simulink subsystem "ButtonStatesRF"  (R2023b) (continued)
== system ButtonStatesRF/ButtonStates/fnCallSubsystem/Function-Call Subsystem23 == (continued)

== system ButtonStatesRF/ButtonStates/fnCallSubsystem/Function-Call Subsystem24 ==
  BLU_FnClkIncDateDig3 : TriggerPort  TriggerType=function-call  IsSimulinkFunction=on  FunctionName=BLU_FnClkIncDateDig3  VariantControl=(inherit)

== system ButtonStatesRF/ButtonStates/fnCallSubsystem/Function-Call Subsystem25 ==
  BLU_FnClkIncDateDig2 : TriggerPort  TriggerType=function-call  IsSimulinkFunction=on  FunctionName=BLU_FnClkIncDateDig2  VariantControl=(inherit)

== system ButtonStatesRF/ButtonStates/fnCallSubsystem/Function-Call Subsystem26 ==
  BLU_FnClkIncDateDig1 : TriggerPort  TriggerType=function-call  IsSimulinkFunction=on  FunctionName=BLU_FnClkIncDateDig1  VariantControl=(inherit)

== system ButtonStatesRF/ButtonStates/fnCallSubsystem/Function-Call Subsystem27 ==
  BLU_FnClkDecDateDig3 : TriggerPort  TriggerType=function-call  IsSimulinkFunction=on  FunctionName=BLU_FnClkDecDateDig3  VariantControl=(inherit)

== system ButtonStatesRF/ButtonStates/fnCallSubsystem/Function-Call Subsystem28 ==
  BLU_FnClkDecDateDig2 : TriggerPort  TriggerType=function-call  IsSimulinkFunction=on  FunctionName=BLU_FnClkDecDateDig2  VariantControl=(inherit)

== system ButtonStatesRF/ButtonStates/fnCallSubsystem/Function-Call Subsystem29 ==
  BLU_FnClkDecDateDig1 : TriggerPort  TriggerType=function-call  IsSimulinkFunction=on  FunctionName=BLU_FnClkDecDateDig1  VariantControl=(inherit)

== system ButtonStatesRF/ButtonStates/fnCallSubsystem/Function-Call Subsystem3 ==
  BLU_FnRLResetRndTmr : TriggerPort  TriggerType=function-call  IsSimulinkFunction=on  FunctionName=BLU_FnRLResetRndTmr  VariantControl=(inherit)

== system ButtonStatesRF/ButtonStates/fnCallSubsystem/Function-Call Subsystem30 ==
  BLU_FnSetHeatedGrips : TriggerPort  TriggerType=function-call  IsSimulinkFunction=on  FunctionName=BLU_FnSetHeatedGrips  VariantControl=(inherit)
  u : ArgIn  OutDataTypeStr=Enum: eStateName  ArgumentName=u
  HeatedGripsOut : Outport
  wires:
  u/1 -> HeatedGripsOut/1

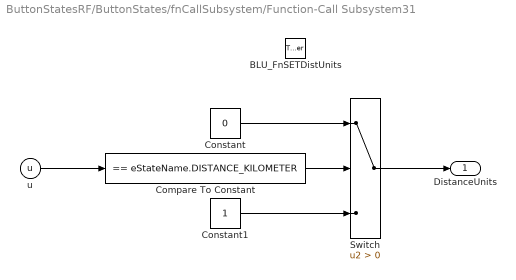
== system ButtonStatesRF/ButtonStates/fnCallSubsystem/Function-Call Subsystem31 ==
  BLU_FnSETDistUnits : TriggerPort  TriggerType=function-call  IsSimulinkFunction=on  FunctionName=BLU_FnSETDistUnits  VariantControl=(inherit)
  Compare To Constant : simulink/Logic and Bit Operations/Compare To Constant  relop===  const=eStateName.DISTANCE_KILOMETER  OutDataTypeStr=boolean
  Constant : Constant  Value=0  OutDataTypeStr=uint8
  Constant1 : Constant  OutDataTypeStr=uint8
  Switch : Switch  Criteria=u2 > Threshold  SaturateOnIntegerOverflow=off  InputSameDT=off
  u : ArgIn  OutDataTypeStr=Enum: eStateName  ArgumentName=u
  DistanceUnits : Outport
  wires:
  Constant1/1 -> Switch/3
  Constant/1 -> Switch/1
  Compare To Constant/1 -> Switch/2
  u/1 -> Compare To Constant/1
  Switch/1 -> DistanceUnits/1

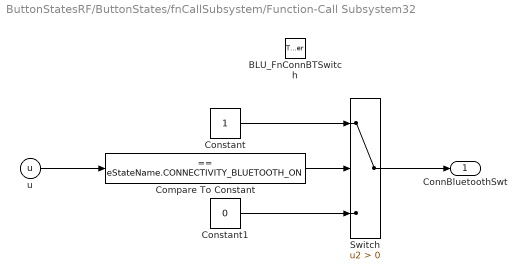
== system ButtonStatesRF/ButtonStates/fnCallSubsystem/Function-Call Subsystem32 ==
  BLU_FnConnBTSwitch : TriggerPort  TriggerType=function-call  IsSimulinkFunction=on  FunctionName=BLU_FnConnBTSwitch  VariantControl=(inherit)
  Compare To Constant : simulink/Logic and Bit Operations/Compare To Constant  relop===  const=eStateName.CONNECTIVITY_BLUETOOTH_ON  OutDataTypeStr=boolean
  Constant : Constant  OutDataTypeStr=uint8
  Constant1 : Constant  Value=0  OutDataTypeStr=uint8
  Switch : Switch  Criteria=u2 > Threshold  SaturateOnIntegerOverflow=off  InputSameDT=off
  u : ArgIn  OutDataTypeStr=Enum: eStateName  ArgumentName=u
  ConnBluetoothSwt : Outport
  wires:
  Switch/1 -> ConnBluetoothSwt/1
  u/1 -> Compare To Constant/1
  Compare To Constant/1 -> Switch/2
  Constant/1 -> Switch/1
  Constant1/1 -> Switch/3

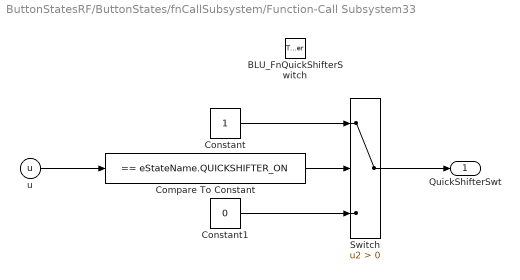
== system ButtonStatesRF/ButtonStates/fnCallSubsystem/Function-Call Subsystem33 ==
  BLU_FnQuickShifterSwitch : TriggerPort  TriggerType=function-call  IsSimulinkFunction=on  FunctionName=BLU_FnQuickShifterSwitch  VariantControl=(inherit)
  Compare To Constant : simulink/Logic and Bit Operations/Compare To Constant  relop===  const=eStateName.QUICKSHIFTER_ON  OutDataTypeStr=boolean
  Constant : Constant  OutDataTypeStr=uint8
  Constant1 : Constant  Value=0  OutDataTypeStr=uint8
  Switch : Switch  Criteria=u2 > Threshold  SaturateOnIntegerOverflow=off  InputSameDT=off
  u : ArgIn  OutDataTypeStr=Enum: eStateName  ArgumentName=u
  QuickShifterSwt : Outport
  wires:
  Constant1/1 -> Switch/3
  Constant/1 -> Switch/1
  Compare To Constant/1 -> Switch/2
  u/1 -> Compare To Constant/1
  Switch/1 -> QuickShifterSwt/1

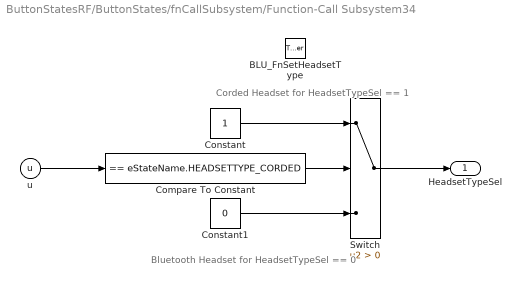
== system ButtonStatesRF/ButtonStates/fnCallSubsystem/Function-Call Subsystem34 ==
  note: Corded Headset for HeadsetTypeSel == 1
  note: Bluetooth Headset for HeadsetTypeSel == 0
  BLU_FnSetHeadsetType : TriggerPort  TriggerType=function-call  IsSimulinkFunction=on  FunctionName=BLU_FnSetHeadsetType  VariantControl=(inherit)
  Compare To Constant : simulink/Logic and Bit Operations/Compare To Constant  relop===  const=eStateName.HEADSETTYPE_CORDED  OutDataTypeStr=boolean
  Constant : Constant  OutDataTypeStr=uint8
  Constant1 : Constant  Value=0  OutDataTypeStr=uint8
  Switch : Switch  Criteria=u2 > Threshold  SaturateOnIntegerOverflow=off  InputSameDT=off
  u : ArgIn  OutDataTypeStr=Enum: eStateName  ArgumentName=u
  HeadsetTypeSel : Outport
  wires:
  Switch/1 -> HeadsetTypeSel/1
  u/1 -> Compare To Constant/1
  Compare To Constant/1 -> Switch/2
  Constant/1 -> Switch/1
  Constant1/1 -> Switch/3

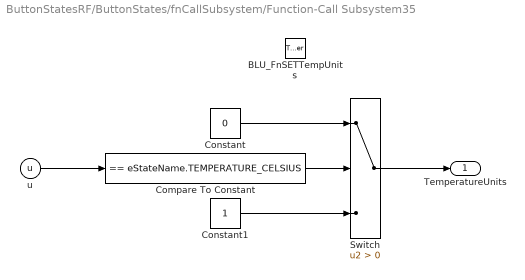
== system ButtonStatesRF/ButtonStates/fnCallSubsystem/Function-Call Subsystem35 ==
  BLU_FnSETTempUnits : TriggerPort  TriggerType=function-call  IsSimulinkFunction=on  FunctionName=BLU_FnSETTempUnits  VariantControl=(inherit)
  Compare To Constant : simulink/Logic and Bit Operations/Compare To Constant  relop===  const=eStateName.TEMPERATURE_CELSIUS  OutDataTypeStr=boolean
  Constant : Constant  Value=0  OutDataTypeStr=uint8
  Constant1 : Constant  OutDataTypeStr=uint8
  Switch : Switch  Criteria=u2 > Threshold  SaturateOnIntegerOverflow=off  InputSameDT=off
  u : ArgIn  OutDataTypeStr=Enum: eStateName  ArgumentName=u
  TemperatureUnits : Outport
  wires:
  Switch/1 -> TemperatureUnits/1
  u/1 -> Compare To Constant/1
  Compare To Constant/1 -> Switch/2
  Constant/1 -> Switch/1
  Constant1/1 -> Switch/3

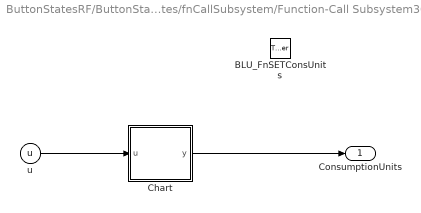
== system ButtonStatesRF/ButtonStates/fnCallSubsystem/Function-Call Subsystem36 ==
  BLU_FnSETConsUnits : TriggerPort  TriggerType=function-call  IsSimulinkFunction=on  FunctionName=BLU_FnSETConsUnits  VariantControl=(inherit)
  Chart : Stateflow chart  TreatAsAtomicUnit=on
  u : ArgIn  OutDataTypeStr=Enum: eStateName  ArgumentName=u
  ConsumptionUnits : Outport
  wires:
  u/1 -> Chart/1(u)
  Chart/1(y) -> ConsumptionUnits/1

== system ButtonStatesRF/ButtonStates/fnCallSubsystem/Function-Call Subsystem37 ==
  BLU_FnIncSrvcDistKM : TriggerPort  TriggerType=function-call  IsSimulinkFunction=on  FunctionName=BLU_FnIncSrvcDistKM  VariantControl=(inherit)

== system ButtonStatesRF/ButtonStates/fnCallSubsystem/Function-Call Subsystem38 ==
  BLU_FnDecSrvcDistKM : TriggerPort  TriggerType=function-call  IsSimulinkFunction=on  FunctionName=BLU_FnDecSrvcDistKM  VariantControl=(inherit)

== system ButtonStatesRF/ButtonStates/fnCallSubsystem/Function-Call Subsystem39 ==
  BLU_FnIncSrvcDistMI : TriggerPort  TriggerType=function-call  IsSimulinkFunction=on  FunctionName=BLU_FnIncSrvcDistMI  VariantControl=(inherit)

== system ButtonStatesRF/ButtonStates/fnCallSubsystem/Function-Call Subsystem4 ==
  BLU_FnTIResetTrip1 : TriggerPort  TriggerType=function-call  IsSimulinkFunction=on  FunctionName=BLU_FnTIResetTrip1  VariantControl=(inherit)

== system ButtonStatesRF/ButtonStates/fnCallSubsystem/Function-Call Subsystem40 ==
  BLU_FnDecSrvcDistMI : TriggerPort  TriggerType=function-call  IsSimulinkFunction=on  FunctionName=BLU_FnDecSrvcDistMI  VariantControl=(inherit)

== system ButtonStatesRF/ButtonStates/fnCallSubsystem/Function-Call Subsystem41 ==
  BLU_FnDecSrvcMonths : TriggerPort  TriggerType=function-call  IsSimulinkFunction=on  FunctionName=BLU_FnDecSrvcMonths  VariantControl=(inherit)

== system ButtonStatesRF/ButtonStates/fnCallSubsystem/Function-Call Subsystem42 ==
  BLU_FnIncSrvcMonths : TriggerPort  TriggerType=function-call  IsSimulinkFunction=on  FunctionName=BLU_FnIncSrvcMonths  VariantControl=(inherit)

== system ButtonStatesRF/ButtonStates/fnCallSubsystem/Function-Call Subsystem43 ==
  BLU_FnSaveSrvcChanges : TriggerPort  TriggerType=function-call  IsSimulinkFunction=on  FunctionName=BLU_FnSaveSrvcChanges  VariantControl=(inherit)

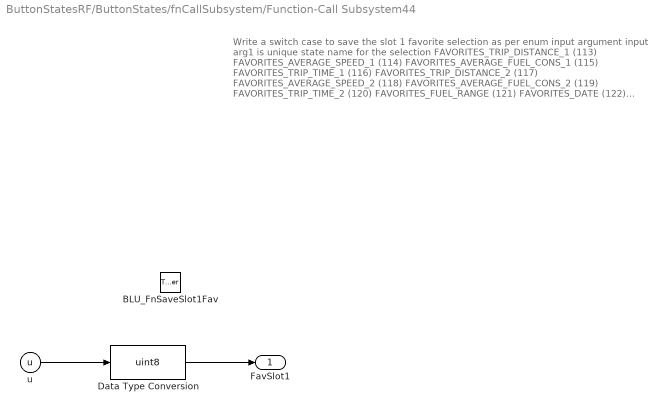
== system ButtonStatesRF/ButtonStates/fnCallSubsystem/Function-Call Subsystem44 ==
  note: Write a switch case to save the slot 1 favorite selection as per enum input argument
      | input arg1 is unique state name for the selection
      | FAVORITES_TRIP_DISTANCE_1 (113)
      | FAVORITES_AVERAGE_SPEED_1 (114)
      | FAVORITES_AVERAGE_FUEL_CONS_1 (115)
      | FAVORITES_TRIP_TIME_1 (116)
      | FAVORITES_TRIP_DISTANCE_2 (117)
      | FAVORITES_AVERAGE_SPEED_2 (118)
      | FAVORITES_AVERAGE_FUEL_CONS_2 (119)
      | FAVORITES_TRIP_TIME_2 (120)
      | FAVORITES_FUEL_RANGE (121)
      | FAVORITES_DATE (122)
      | FAVORITES_BATTERY (123)
      | FAVORITES_WATER_TEMPERATURE (124)
      | FAVORITES_ODOMETER (125)
      | FAVORITES_SLOT_1 (126)
      | FAVORITES_SLOT_2 (127)
      | FAVORITES_SLOT_3 (128)
      | FAVORITES_SLOT_4 (129)
  BLU_FnSaveSlot1Fav : TriggerPort  TriggerType=function-call  IsSimulinkFunction=on  FunctionName=BLU_FnSaveSlot1Fav  VariantControl=(inherit)
  Data Type Conversion : DataTypeConversion  OutDataTypeStr=uint8  SaturateOnIntegerOverflow=off  RndMeth=Floor
  u : ArgIn  ArgumentName=u
  FavSlot1 : Outport  OutDataTypeStr=uint8
  wires:
  u/1 -> Data Type Conversion/1
  Data Type Conversion/1 -> FavSlot1/1

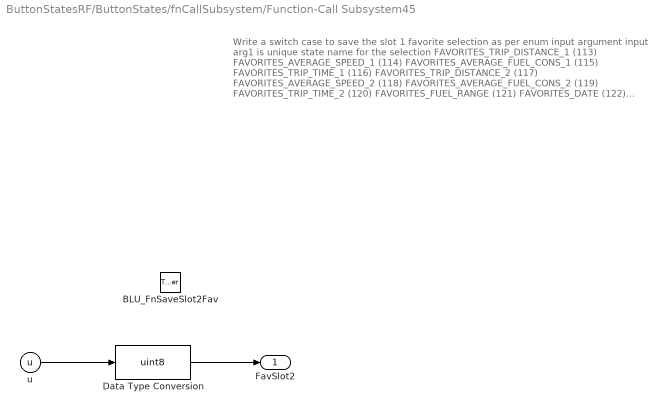
== system ButtonStatesRF/ButtonStates/fnCallSubsystem/Function-Call Subsystem45 ==
  note: Write a switch case to save the slot 1 favorite selection as per enum input argument
      | input arg1 is unique state name for the selection
      | FAVORITES_TRIP_DISTANCE_1 (113)
      | FAVORITES_AVERAGE_SPEED_1 (114)
      | FAVORITES_AVERAGE_FUEL_CONS_1 (115)
      | FAVORITES_TRIP_TIME_1 (116)
      | FAVORITES_TRIP_DISTANCE_2 (117)
      | FAVORITES_AVERAGE_SPEED_2 (118)
      | FAVORITES_AVERAGE_FUEL_CONS_2 (119)
      | FAVORITES_TRIP_TIME_2 (120)
      | FAVORITES_FUEL_RANGE (121)
      | FAVORITES_DATE (122)
      | FAVORITES_BATTERY (123)
      | FAVORITES_WATER_TEMPERATURE (124)
      | FAVORITES_ODOMETER (125)
      | FAVORITES_SLOT_1 (126)
      | FAVORITES_SLOT_2 (127)
      | FAVORITES_SLOT_3 (128)
      | FAVORITES_SLOT_4 (129)
  BLU_FnSaveSlot2Fav : TriggerPort  TriggerType=function-call  IsSimulinkFunction=on  FunctionName=BLU_FnSaveSlot2Fav  VariantControl=(inherit)
  Data Type Conversion : DataTypeConversion  OutDataTypeStr=uint8  SaturateOnIntegerOverflow=off  RndMeth=Floor
  u : ArgIn  ArgumentName=u
  FavSlot2 : Outport  OutDataTypeStr=uint8
  wires:
  u/1 -> Data Type Conversion/1
  Data Type Conversion/1 -> FavSlot2/1

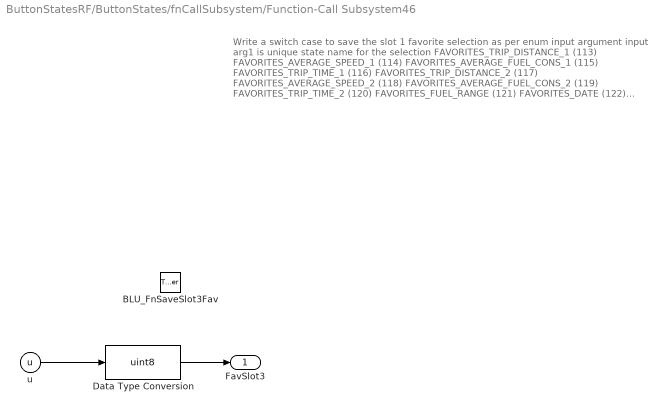
== system ButtonStatesRF/ButtonStates/fnCallSubsystem/Function-Call Subsystem46 ==
  note: Write a switch case to save the slot 1 favorite selection as per enum input argument
      | input arg1 is unique state name for the selection
      | FAVORITES_TRIP_DISTANCE_1 (113)
      | FAVORITES_AVERAGE_SPEED_1 (114)
      | FAVORITES_AVERAGE_FUEL_CONS_1 (115)
      | FAVORITES_TRIP_TIME_1 (116)
      | FAVORITES_TRIP_DISTANCE_2 (117)
      | FAVORITES_AVERAGE_SPEED_2 (118)
      | FAVORITES_AVERAGE_FUEL_CONS_2 (119)
      | FAVORITES_TRIP_TIME_2 (120)
      | FAVORITES_FUEL_RANGE (121)
      | FAVORITES_DATE (122)
      | FAVORITES_BATTERY (123)
      | FAVORITES_WATER_TEMPERATURE (124)
      | FAVORITES_ODOMETER (125)
      | FAVORITES_SLOT_1 (126)
      | FAVORITES_SLOT_2 (127)
      | FAVORITES_SLOT_3 (128)
      | FAVORITES_SLOT_4 (129)
  BLU_FnSaveSlot3Fav : TriggerPort  TriggerType=function-call  IsSimulinkFunction=on  FunctionName=BLU_FnSaveSlot3Fav  VariantControl=(inherit)
  Data Type Conversion : DataTypeConversion  OutDataTypeStr=uint8  SaturateOnIntegerOverflow=off  RndMeth=Floor
  u : ArgIn  ArgumentName=u
  FavSlot3 : Outport  OutDataTypeStr=uint8
  wires:
  u/1 -> Data Type Conversion/1
  Data Type Conversion/1 -> FavSlot3/1

== system ButtonStatesRF/ButtonStates/fnCallSubsystem/Function-Call Subsystem47 ==
  note: Write a switch case to save the slot 1 favorite selection as per enum input argument
      | input arg1 is unique state name for the selection
      | FAVORITES_TRIP_DISTANCE_1 (113)
      | FAVORITES_AVERAGE_SPEED_1 (114)
      | FAVORITES_AVERAGE_FUEL_CONS_1 (115)
      | FAVORITES_TRIP_TIME_1 (116)
      | FAVORITES_TRIP_DISTANCE_2 (117)
      | FAVORITES_AVERAGE_SPEED_2 (118)
      | FAVORITES_AVERAGE_FUEL_CONS_2 (119)
      | FAVORITES_TRIP_TIME_2 (120)
      | FAVORITES_FUEL_RANGE (121)
      | FAVORITES_DATE (122)
      | FAVORITES_BATTERY (123)
      | FAVORITES_WATER_TEMPERATURE (124)
      | FAVORITES_ODOMETER (125)
      | FAVORITES_SLOT_1 (126)
      | FAVORITES_SLOT_2 (127)
      | FAVORITES_SLOT_3 (128)
      | FAVORITES_SLOT_4 (129)
  BLU_FnSaveSlot4Fav : TriggerPort  TriggerType=function-call  IsSimulinkFunction=on  FunctionName=BLU_FnSaveSlot4Fav  VariantControl=(inherit)
  Data Type Conversion : DataTypeConversion  OutDataTypeStr=uint8  SaturateOnIntegerOverflow=off  RndMeth=Floor
  u : ArgIn  ArgumentName=u
  FavSlot4 : Outport  OutDataTypeStr=uint8
  wires:
  u/1 -> Data Type Conversion/1
  Data Type Conversion/1 -> FavSlot4/1

== system ButtonStatesRF/ButtonStates/fnCallSubsystem/Function-Call Subsystem48 ==
  BLU_FnLaunchControlON : TriggerPort  TriggerType=function-call  IsSimulinkFunction=on  FunctionName=BLU_FnLaunchControlON  VariantControl=(inherit)

== system ButtonStatesRF/ButtonStates/fnCallSubsystem/Function-Call Subsystem49 ==
  BLU_FnLaunchControlOFF : TriggerPort  TriggerType=function-call  IsSimulinkFunction=on  FunctionName=BLU_FnLaunchControlOFF  VariantControl=(inherit)

== system ButtonStatesRF/ButtonStates/fnCallSubsystem/Function-Call Subsystem5 ==
  BLU_FnTIResetTrip2 : TriggerPort  TriggerType=function-call  IsSimulinkFunction=on  FunctionName=BLU_FnTIResetTrip2  VariantControl=(inherit)

== system ButtonStatesRF/ButtonStates/fnCallSubsystem/Function-Call Subsystem50 ==
  BLU_FnShiftLightOFF : TriggerPort  TriggerType=function-call  IsSimulinkFunction=on  FunctionName=BLU_FnShiftLightOFF  VariantControl=(inherit)

== system ButtonStatesRF/ButtonStates/fnCallSubsystem/Function-Call Subsystem51 ==
  BLU_FnShiftLightON : TriggerPort  TriggerType=function-call  IsSimulinkFunction=on  FunctionName=BLU_FnShiftLightON  VariantControl=(inherit)

== system ButtonStatesRF/ButtonStates/fnCallSubsystem/Function-Call Subsystem52 ==
  BLU_FnSetQuickSelectorUP : TriggerPort  TriggerType=function-call  IsSimulinkFunction=on  FunctionName=BLU_FnSetQuickSelectorUP  VariantControl=(inherit)
  Terminator : Terminator
  u : ArgIn  ArgumentName=u
  wires:
  u/1 -> Terminator/1

== system ButtonStatesRF/ButtonStates/fnCallSubsystem/Function-Call Subsystem53 ==
  BLU_FnSetQuickSelectorDOWN : TriggerPort  TriggerType=function-call  IsSimulinkFunction=on  FunctionName=BLU_FnSetQuickSelectorDOWN  VariantControl=(inherit)
  Terminator : Terminator
  u : ArgIn  ArgumentName=u
  wires:
  u/1 -> Terminator/1

== system ButtonStatesRF/ButtonStates/fnCallSubsystem/Function-Call Subsystem54 ==
  BLU_FnNavigationStatus : TriggerPort  TriggerType=function-call  IsSimulinkFunction=on  FunctionName=BLU_FnNavigationStatus  VariantControl=(inherit)
  Terminator : Terminator
  u : ArgIn  ArgumentName=u
  wires:
  u/1 -> Terminator/1

== system ButtonStatesRF/ButtonStates/fnCallSubsystem/Function-Call Subsystem55 ==
  BLU_FnSETLanguage : TriggerPort  TriggerType=function-call  IsSimulinkFunction=on  FunctionName=BLU_FnSETLanguage  VariantControl=(inherit)
  Terminator : Terminator
  u : ArgIn  OutDataTypeStr=Enum: eStateName  ArgumentName=u
  wires:
  u/1 -> Terminator/1

== system ButtonStatesRF/ButtonStates/fnCallSubsystem/Function-Call Subsystem56 ==
  BLU_FnTISetAsOvwTrip1 : TriggerPort  TriggerType=function-call  IsSimulinkFunction=on  FunctionName=BLU_FnTISetAsOvwTrip1  VariantControl=(inherit)

== system ButtonStatesRF/ButtonStates/fnCallSubsystem/Function-Call Subsystem57 ==
  BLU_FnTISetAsOvwTrip2 : TriggerPort  TriggerType=function-call  IsSimulinkFunction=on  FunctionName=BLU_FnTISetAsOvwTrip2  VariantControl=(inherit)

== system ButtonStatesRF/ButtonStates/fnCallSubsystem/Function-Call Subsystem58 ==
  BLU_FnClkFrmtTgle : TriggerPort  TriggerType=function-call  IsSimulinkFunction=on  FunctionName=BLU_FnClkFrmtTgle  VariantControl=(inherit)
  Terminator : Terminator
  u : ArgIn  OutDataTypeStr=Enum: eStateName  ArgumentName=u
  wires:
  u/1 -> Terminator/1

== system ButtonStatesRF/ButtonStates/fnCallSubsystem/Function-Call Subsystem59 ==
  BLU_FnDateFrmtTgle : TriggerPort  TriggerType=function-call  IsSimulinkFunction=on  FunctionName=BLU_FnDateFrmtTgle  VariantControl=(inherit)
  Terminator : Terminator
  u : ArgIn  OutDataTypeStr=Enum: eStateName  ArgumentName=u
  wires:
  u/1 -> Terminator/1

== system ButtonStatesRF/ButtonStates/fnCallSubsystem/Function-Call Subsystem6 ==
  BLU_FnBIWarningsUp : TriggerPort  TriggerType=function-call  IsSimulinkFunction=on  FunctionName=BLU_FnBIWarningsUp  VariantControl=(inherit)

== system ButtonStatesRF/ButtonStates/fnCallSubsystem/Function-Call Subsystem60 ==
  BLU_FnSetHeatedGripsMode : TriggerPort  TriggerType=function-call  IsSimulinkFunction=on  FunctionName=BLU_FnSetHeatedGripsMode  VariantControl=(inherit)
  Terminator : Terminator
  u : ArgIn  OutDataTypeStr=Enum: eStateName  ArgumentName=u
  wires:
  u/1 -> Terminator/1

== system ButtonStatesRF/ButtonStates/fnCallSubsystem/Function-Call Subsystem61 ==
  BLU_FnIncomingCallAnswer : TriggerPort  TriggerType=function-call  IsSimulinkFunction=on  FunctionName=BLU_FnIncomingCallAnswer  VariantControl=(inherit)

== system ButtonStatesRF/ButtonStates/fnCallSubsystem/Function-Call Subsystem62 ==
  BLU_FnIncomingCallReject : TriggerPort  TriggerType=function-call  IsSimulinkFunction=on  FunctionName=BLU_FnIncomingCallReject  VariantControl=(inherit)

== system ButtonStatesRF/ButtonStates/fnCallSubsystem/Function-Call Subsystem63 ==
  BLU_FnCallVolInc : TriggerPort  TriggerType=function-call  IsSimulinkFunction=on  FunctionName=BLU_FnCallVolInc  VariantControl=(inherit)

== system ButtonStatesRF/ButtonStates/fnCallSubsystem/Function-Call Subsystem64 ==
  BLU_FnCallVolDec : TriggerPort  TriggerType=function-call  IsSimulinkFunction=on  FunctionName=BLU_FnCallVolDec  VariantControl=(inherit)

== system ButtonStatesRF/ButtonStates/fnCallSubsystem/Function-Call Subsystem65 ==
  BLU_FnEndCall : TriggerPort  TriggerType=function-call  IsSimulinkFunction=on  FunctionName=BLU_FnEndCall  VariantControl=(inherit)

== system ButtonStatesRF/ButtonStates/fnCallSubsystem/Function-Call Subsystem66 ==
  BLU_FnBTPhoneDvcUp : TriggerPort  TriggerType=function-call  IsSimulinkFunction=on  FunctionName=BLU_FnBTPhoneDvcUp  VariantControl=(inherit)

== system ButtonStatesRF/ButtonStates/fnCallSubsystem/Function-Call Subsystem67 ==
  BLU_FnBTPhoneDvcDwn : TriggerPort  TriggerType=function-call  IsSimulinkFunction=on  FunctionName=BLU_FnBTPhoneDvcDwn  VariantControl=(inherit)

== system ButtonStatesRF/ButtonStates/fnCallSubsystem/Function-Call Subsystem68 ==
  BLU_FnBTHeadsetDvcUp : TriggerPort  TriggerType=function-call  IsSimulinkFunction=on  FunctionName=BLU_FnBTHeadsetDvcUp  VariantControl=(inherit)

== system ButtonStatesRF/ButtonStates/fnCallSubsystem/Function-Call Subsystem69 ==
  BLU_FnBTHeadsetDvcDwn : TriggerPort  TriggerType=function-call  IsSimulinkFunction=on  FunctionName=BLU_FnBTHeadsetDvcDwn  VariantControl=(inherit)

== system ButtonStatesRF/ButtonStates/fnCallSubsystem/Function-Call Subsystem7 ==
  BLU_FnBIWarningsDwn : TriggerPort  TriggerType=function-call  IsSimulinkFunction=on  FunctionName=BLU_FnBIWarningsDwn  VariantControl=(inherit)

== system ButtonStatesRF/ButtonStates/fnCallSubsystem/Function-Call Subsystem70 ==
  BLU_FnBTPhoneDvcDel : TriggerPort  TriggerType=function-call  IsSimulinkFunction=on  FunctionName=BLU_FnBTPhoneDvcDel  VariantControl=(inherit)

== system ButtonStatesRF/ButtonStates/fnCallSubsystem/Function-Call Subsystem71 ==
  BLU_FnBTHeadsetDvcDel : TriggerPort  TriggerType=function-call  IsSimulinkFunction=on  FunctionName=BLU_FnBTHeadsetDvcDel  VariantControl=(inherit)

== system ButtonStatesRF/ButtonStates/fnCallSubsystem/Function-Call Subsystem72 ==
  BLU_FnBTPhonePassKeyChkd : TriggerPort  TriggerType=function-call  IsSimulinkFunction=on  FunctionName=BLU_FnBTPhonePassKeyChkd  VariantControl=(inherit)

== system ButtonStatesRF/ButtonStates/fnCallSubsystem/Function-Call Subsystem73 ==
  BLU_FnBTPhonePairReq : TriggerPort  TriggerType=function-call  IsSimulinkFunction=on  FunctionName=BLU_FnBTPhonePairReq  VariantControl=(inherit)

== system ButtonStatesRF/ButtonStates/fnCallSubsystem/Function-Call Subsystem74 ==
  BLU_FnBTHeadsetPairReq : TriggerPort  TriggerType=function-call  IsSimulinkFunction=on  FunctionName=BLU_FnBTHeadsetPairReq  VariantControl=(inherit)

== system ButtonStatesRF/ButtonStates/fnCallSubsystem/Function-Call Subsystem75 ==
  BLU_FnCnctvtyCnfrmAppReg : TriggerPort  TriggerType=function-call  IsSimulinkFunction=on  FunctionName=BLU_FnCnctvtyCnfrmAppReg  VariantControl=(inherit)
  Terminator : Terminator
  u : ArgIn  ArgumentName=u
  wires:
  u/1 -> Terminator/1

== system ButtonStatesRF/ButtonStates/fnCallSubsystem/Function-Call Subsystem76 ==
  BLU_FnHdstSelCord : TriggerPort  TriggerType=function-call  IsSimulinkFunction=on  FunctionName=BLU_FnHdstSelCord  VariantControl=(inherit)

== system ButtonStatesRF/ButtonStates/fnCallSubsystem/Function-Call Subsystem77 ==
  BLU_FnHdstSelBT : TriggerPort  TriggerType=function-call  IsSimulinkFunction=on  FunctionName=BLU_FnHdstSelBT  VariantControl=(inherit)

== system ButtonStatesRF/ButtonStates/fnCallSubsystem/Function-Call Subsystem78 ==
  BLU_FnActvCallEXIT : TriggerPort  TriggerType=function-call  IsSimulinkFunction=on  FunctionName=BLU_FnActvCallEXIT  VariantControl=(inherit)

== system ButtonStatesRF/ButtonStates/fnCallSubsystem/Function-Call Subsystem79 ==
  BLU_FnLapTmrScrllUP : TriggerPort  TriggerType=function-call  IsSimulinkFunction=on  FunctionName=BLU_FnLapTmrScrllUP  VariantControl=(inherit)

== system ButtonStatesRF/ButtonStates/fnCallSubsystem/Function-Call Subsystem8 ==
  BLU_FnCCSwtONSpdLmtr : TriggerPort  TriggerType=function-call  IsSimulinkFunction=on  FunctionName=BLU_FnCCSwtONSpdLmtr  VariantControl=(inherit)

== system ButtonStatesRF/ButtonStates/fnCallSubsystem/Function-Call Subsystem80 ==
  BLU_FnActvCallSET : TriggerPort  TriggerType=function-call  IsSimulinkFunction=on  FunctionName=BLU_FnActvCallSET  VariantControl=(inherit)

== system ButtonStatesRF/ButtonStates/fnCallSubsystem/Function-Call Subsystem81 ==
  BLU_FnLapTmrScrllDOWN : TriggerPort  TriggerType=function-call  IsSimulinkFunction=on  FunctionName=BLU_FnLapTmrScrllDOWN  VariantControl=(inherit)

== system ButtonStatesRF/ButtonStates/fnCallSubsystem/Function-Call Subsystem82 ==
  BLU_FnClosePopUp : TriggerPort  TriggerType=function-call  IsSimulinkFunction=on  FunctionName=BLU_FnClosePopUp  VariantControl=(inherit)

== system ButtonStatesRF/ButtonStates/fnCallSubsystem/Function-Call Subsystem9 ==
  BLU_FnCCSwtOFFSpdLmtr : TriggerPort  TriggerType=function-call  IsSimulinkFunction=on  FunctionName=BLU_FnCCSwtOFFSpdLmtr  VariantControl=(inherit)

== stateflow chart ButtonStatesRF/ButtonStates/fnCallSubsystem/Function-Call Subsystem36/Chart ==
  (default) -> (junction 1)
  (junction 1) -> (junction 3) [order 1] : [u == eStateName.CONSUMPTION_LTR_PER_100KM]
  (junction 3) -> (junction 5) : {y=0;}
  (junction 1) -> (junction 7) [order 2]
  (junction 5) -> (junction 11)
  (junction 7) -> (junction 9) [order 1] : [u == eStateName.CONSUMPTION_KM_PER_LTR]
  (junction 9) -> (junction 11) : {y=1;}
  (junction 7) -> (junction 14) [order 2]
  (junction 11) -> (junction 18)
  (junction 14) -> (junction 16) [order 1] : [u == eStateName.CONSUMPTION_USG_PER_100MI]
  (junction 16) -> (junction 18) : {y=2;}
  (junction 14) -> (junction 21) [order 2]
  (junction 18) -> (junction 25)
  (junction 23) -> (junction 25) : {y=3; }
  (junction 21) -> (junction 23) [order 1] : [u == CONSUMPTION_MI_PER_USG]
  (junction 25) -> (junction 32)
  (junction 21) -> (junction 28) [order 2]
  (junction 28) -> (junction 30) [order 1] : [u==CONSUMPTION_MI_PER_LTR]
  (junction 30) -> (junction 32) : {y=4;}
  (junction 28) -> (junction 35) [order 2]
  (junction 32) -> (junction 39)
  (junction 37) -> (junction 39) : {y=5;}
  (junction 35) -> (junction 37) [order 1] : [u == CONSUMPTION_UKG_PER_100MI]
  (junction 39) -> (junction 46)
  (junction 35) -> (junction 42) [order 2]
  (junction 44) -> (junction 46) : {y=6;}
  (junction 42) -> (junction 44) [order 1] : [u==CONSUMPTION_MI_PER_UKG]
  (junction 46) -> (junction 52)
  (junction 42) -> (junction 50) [order 2]
  (junction 52) -> (junction 50)
  data: y output uint16; u input
